simulink library "lib_all"  (Simulink 7.6)
settings: Solver=ode45  SolverMode=SingleTasking  StartTime=0.0  StopTime=10.0  RelTol=1e-3
values from neighbouring files (symbols this model uses):
  map_lib_03 = [6.8679 7.0159 7.4571 8.1849 9.1892 10.456 11.97 13.71 15.655 17.781 20.063 22.474 ... (803 elements, 73x11)]    [scr_simdata.mat]    (also in scr_simdata_all.mat)
  map_lib_06 = [2.5784 2.6727 2.9588 3.4453 4.1444 5.068 6.226 7.6242 9.2634 11.139 13.24 15.552 ... (803 elements, 73x11)]    [scr_simdata.mat]    (also in scr_simdata_all.mat)
  map_lib_09 = [0.0025784 0.0026727 0.0029588 0.0034453 0.0041444 0.005068 0.006226 0.0076242 0.0092634 0.011139 0.01324 0.015552 ... (803 elements, 73x11)]    [scr_simdata.mat]    (also in scr_simdata_all.mat)
  map_lib_10 = [2.74 2.75 2.76 2.77 2.78 2.79 2.8 2.81 2.82 2.83 2.84 2.85 ... (81 elements, 1x81)]    [scr_simdata.mat]    (also in scr_simdata_all.mat)
  map_lib_11 = [1.1983 1.2027 1.207 1.2113 1.2156 1.2198 1.2241 1.2283 1.2326 1.2368 1.241 1.2451 ... (81 elements, 1x81)]    [scr_simdata.mat]    (also in scr_simdata_all.mat)
  map_lib_12 = [2.74 2.75 2.76 2.77 2.78 2.79 2.8 2.81 2.82 2.83 2.84 2.85 ... (81 elements, 1x81)]    [scr_simdata.mat]    (also in scr_simdata_all.mat)
  map_lib_13 = [1.74 1.75 1.76 1.77 1.78 1.79 1.8 1.81 1.82 1.83 1.84 1.85 ... (81 elements, 1x81)]    [scr_simdata.mat]    (also in scr_simdata_all.mat)
  map_lib_16 = [0.00048828125 1.6159 1.8962 2.0821 2.2251 2.3426 2.4433 2.5318 2.611 2.683 2.749 2.8101 ... (1419 elements, 129x11)]    [scr_simdata.mat]    (also in scr_simdata_all.mat)
  map_mr2_01 = [0 800 1600 2400 3200 4000]    [scr_simdata.mat]    (also in scr_simdata_all.mat)
  map_mr2_02 = [1.32 1.32 1.33 1.34 1.36 1.38]    [scr_simdata.mat]    (also in scr_simdata_all.mat)
  map_mr2_03 = [0 800 1600 2400 3200 4000]    [scr_simdata.mat]    (also in scr_simdata_all.mat)
  map_mr2_04 = [1.32 1.32 1.33 1.34 1.36 1.38]    [scr_simdata.mat]    (also in scr_simdata_all.mat)
  map_mr2_05 = [0 800 1600 2400 3200 4000]    [scr_simdata.mat]    (also in scr_simdata_all.mat)
  map_mr2_06 = [1.32 1.32 1.33 1.34 1.36 1.38]    [scr_simdata.mat]    (also in scr_simdata_all.mat)
  Parameter1 = 6    [scr_simdata.mat]    (also in scr_simdata_all.mat)
  Parameter101 = 30    [scr_simdata.mat]    (also in scr_simdata_all.mat)
  Parameter102 = 12    [scr_simdata.mat]    (also in scr_simdata_all.mat)
  Parameter103 = -240    [scr_simdata.mat]    (also in scr_simdata_all.mat)
  Parameter108 = 48    [scr_simdata.mat]    (also in scr_simdata_all.mat)
  Parameter120 = 16    [scr_simdata.mat]    (also in scr_simdata_all.mat)
  Parameter121 = 87    [scr_simdata.mat]    (also in scr_simdata_all.mat)
  Parameter123 = 78    [scr_simdata.mat]    (also in scr_simdata_all.mat)
  Parameter124 = 60    [scr_simdata.mat]    (also in scr_simdata_all.mat)
  Parameter125 = -480    [scr_simdata.mat]    (also in scr_simdata_all.mat)
  Parameter130 = 96    [scr_simdata.mat]    (also in scr_simdata_all.mat)
  Parameter131 = 3    [scr_simdata.mat]    (also in scr_simdata_all.mat)
  Parameter144 = 3    [scr_simdata.mat]    (also in scr_simdata_all.mat)
  Parameter18 = 128    [scr_simdata.mat]    (also in scr_simdata_all.mat)
  Parameter19 = 16    [scr_simdata.mat]    (also in scr_simdata_all.mat)
  Parameter2 = 12    [scr_simdata.mat]    (also in scr_simdata_all.mat)
  Parameter20 = 7    [scr_simdata.mat]    (also in scr_simdata_all.mat)
  Parameter21 = 4    [scr_simdata.mat]    (also in scr_simdata_all.mat)
  Parameter3 = 18    [scr_simdata.mat]    (also in scr_simdata_all.mat)
  Parameter32 = 1    [scr_simdata.mat]    (also in scr_simdata_all.mat)
  Parameter34 = 24    [scr_simdata.mat]    (also in scr_simdata_all.mat)
  Parameter4 = 138    [scr_simdata.mat]    (also in scr_simdata_all.mat)
  Parameter42 = 48    [scr_simdata.mat]    (also in scr_simdata_all.mat)
  Parameter5 = 135    [scr_simdata.mat]    (also in scr_simdata_all.mat)
  Parameter55 = 0    [scr_simdata.mat]    (also in scr_simdata_all.mat)
  Parameter56 = 0    [scr_simdata.mat]    (also in scr_simdata_all.mat)
  Parameter57 = 1    [scr_simdata.mat]    (also in scr_simdata_all.mat)
  Parameter58 = 1    [scr_simdata.mat]    (also in scr_simdata_all.mat)
  Parameter59 = 2    [scr_simdata.mat]    (also in scr_simdata_all.mat)
  Parameter6 = 132    [scr_simdata.mat]    (also in scr_simdata_all.mat)
  Parameter60 = 2    [scr_simdata.mat]    (also in scr_simdata_all.mat)
  Parameter63 = 144    [scr_simdata.mat]    (also in scr_simdata_all.mat)
  Parameter7 = 126    [scr_simdata.mat]    (also in scr_simdata_all.mat)
  Parameter76 = 64    [scr_simdata.mat]    (also in scr_simdata_all.mat)
  Parameter77 = 135    [scr_simdata.mat]    (also in scr_simdata_all.mat)
  Parameter79 = 126    [scr_simdata.mat]    (also in scr_simdata_all.mat)
  Parameter80 = 108    [scr_simdata.mat]    (also in scr_simdata_all.mat)
  Parameter81 = 0    [scr_simdata.mat]    (also in scr_simdata_all.mat)
  Parameter86 = 0    [scr_simdata.mat]    (also in scr_simdata_all.mat)
  Parameter98 = 112    [scr_simdata.mat]    (also in scr_simdata_all.mat)
  Parameter99 = 39    [scr_simdata.mat]    (also in scr_simdata_all.mat)
undefined symbols (not defined in the file or in its folder): cylvolkp_hih_map2h, cylvolkp_map2h, pk1k_map2h
libraries: simulink x2
(part 13 of 36)

== system lib_all/SubSystem176/SubSystem178/SubSystem182/SubSystem236 ==
  note: Text41
  Block535 : Inport
  Block536 : Inport  Port=2
  Block537 : Inport  Port=3
  Block538 : Inport  Port=4
  Block539 : Constant  Value=0  OutDataTypeStr=double  SampleTime=-1
  Block540 : Constant  OutDataTypeStr=double  SampleTime=-1
  Block541 : Constant  Value=0  OutDataTypeStr=double  SampleTime=-1
  Block542 : Gain  Gain=1/5  SaturateOnIntegerOverflow=on  OutDataTypeStr=Inherit: Same as input  ParamDataTypeStr=Inherit: Same as input
      settings: AttributesFormatString=Attribute119
  Block543 : Product  OutDataTypeStr=double  SaturateOnIntegerOverflow=on  RndMeth=Zero  InputSameDT=on
  Block544 : Sum  Inputs=-+  OutDataTypeStr=double  RndMeth=Zero  SaturateOnIntegerOverflow=on  InputSameDT=on
  Block545 : Sum  OutDataTypeStr=double  RndMeth=Zero  SaturateOnIntegerOverflow=on  InputSameDT=on
  Block546 : Sum  OutDataTypeStr=double  RndMeth=Zero  SaturateOnIntegerOverflow=on  InputSameDT=on
  Block547 : Switch  Threshold=0.5  RndMeth=Zero  SaturateOnIntegerOverflow=on  InputSameDT=on
  SubSystem238 : Subsystem
  SubSystem239 : Subsystem
  SubSystem245 : Subsystem
  Block656 : Outport
  wires:
  Block546/1 -> SubSystem245/3(Block577), SubSystem239/2(Block549)
  Block535/1 -> Block546/1
  Block542/1 -> Block545/2
  Block543/1 -> Block542/1
  Block540/1 -> Block546/2
  Block539/1 -> Block547/1
  Block547/1 -> Block656/1
  SubSystem238/1(Out1) -> Block547/2
  Block541/1 -> SubSystem238/2(In2)
  Block537/1 -> SubSystem239/3(Block550)
  Block545/1 -> SubSystem238/1(In1), Block547/3
  SubSystem239/2(Block574) -> Block543/2
  Block544/1 -> Block543/1  "SigLabel14"
  SubSystem245/2(Block655) -> Block544/2
  SubSystem245/1(Block654) -> Block544/1, Block545/1
  Block538/1 -> SubSystem245/2(Block576)
  SubSystem239/1(Block573) -> SubSystem245/1(Block575)
  Block536/1 -> SubSystem239/1(Block548)

== system lib_all/SubSystem176/SubSystem178/SubSystem182/SubSystem289 ==
  note: Text42
  Block657 : Inport
  Block658 : Inport  Port=2
  Block659 : Inport  Port=3
  Block660 : SwitchCase  CaseConditions={1 2 3}
  Block661 : Terminator
  SubSystem290 : Subsystem
      settings: RTWSystemCode=Inline
  SubSystem292 : Subsystem
      settings: RTWSystemCode=Inline
  SubSystem294 : Subsystem
      settings: RTWSystemCode=Inline
  wires:
  Block660/2 -> SubSystem292/Action
  Block660/1 -> SubSystem290/Action
  Block659/1 -> SubSystem292/2(Block666), SubSystem294/2(Block669), SubSystem290/2(Block663)
  Block657/1 -> SubSystem292/1(Block665), SubSystem294/1(Block668), SubSystem290/1(Block662)
  Block658/1 -> Block660/1
  Block660/4 -> Block661/1
  Block660/3 -> SubSystem294/Action

== system lib_all/SubSystem176/SubSystem178/SubSystem182/SubSystem296 ==
  note: Text43
  Block671 : Inport
  Block672 : Inport  Port=2
  Block673 : Inport  Port=3
  Block674 : SwitchCase  CaseConditions={1 2 3}
  Block675 : Terminator
  SubSystem297 : Subsystem
      settings: RTWSystemCode=Inline
  SubSystem299 : Subsystem
      settings: RTWSystemCode=Inline
  SubSystem301 : Subsystem
      settings: RTWSystemCode=Inline
  wires:
  Block672/1 -> Block674/1
  Block671/1 -> SubSystem297/1(Block676), SubSystem299/1(Block679), SubSystem301/1(Block682)
  Block673/1 -> SubSystem297/2(Block677), SubSystem299/2(Block680), SubSystem301/2(Block683)
  Block674/1 -> SubSystem297/Action
  Block674/2 -> SubSystem299/Action
  Block674/4 -> Block675/1
  Block674/3 -> SubSystem301/Action

== system lib_all/SubSystem176/SubSystem303/SubSystem305/SubSystem306 ==
  note: ***編集禁止***
  N : Inport
  For_loop : Stateflow chart  TreatAsAtomicUnit=on
  action : Outport
  i : Outport  Port=2
  wires:
  For_loop/2(function_call) -> action/1
  N/1 -> For_loop/1(N_Loop)
  For_loop/1(idx) -> i/1

== system lib_all/SubSystem176/SubSystem303/SubSystem305/SubSystem308 ==
  Block695 : Inport
  Block696 : Inport  Port=2
  Block697 : Inport  Port=3
  Block698 : Constant  Value=Parameter21  OutDataTypeStr=double  SampleTime=-1
  Block699 : Constant  Value=2  OutDataTypeStr=double  SampleTime=-1
  Block700 : Constant  Value=0  OutDataTypeStr=double  SampleTime=-1
  Block701 : Math  Operator=pow  SaturateOnIntegerOverflow=on
  Block702 : Product  Inputs=*/  OutDataTypeStr=double  SaturateOnIntegerOverflow=on  RndMeth=Zero  InputSameDT=on
  Block703 : Product  Inputs=*/  OutDataTypeStr=double  SaturateOnIntegerOverflow=on  RndMeth=Zero  InputSameDT=on
  Block704 : Sum  Inputs=-+  OutDataTypeStr=double  RndMeth=Zero  SaturateOnIntegerOverflow=on  InputSameDT=on
  SubSystem309 : Subsystem
  SubSystem310 : Subsystem  Priority=2  TreatAsAtomicUnit=on  mask(***ATC.DSWVEC***){bd_version=1.01.A, r0=DSM377, r1=-1, r2=, r3=, r4=, r5=, r6=, r7=, r8=, r9=, c0=, c1=, c2=, c3=, c4=, c5=, c6=, c7=, c8=, c9=}
      mask prompts: bd_version "Version"
      mask help: これはAutoCからのブロックです．編集禁止．
      settings: AttributesFormatString=Attribute141  OpenFcn=warndlg('変更禁止!');
      mask Help: web(ui_blockhelpmng(gcb));
      mask Display: disp('ATC.DSRVEC')
  SubSystem311 : Subsystem  Priority=3  TreatAsAtomicUnit=on  mask(***ATC.DSWVEC***){bd_version=1.01.A, r0=DSM379, r1=-1, r2=, r3=, r4=, r5=, r6=, r7=, r8=, r9=, c0=, c1=, c2=, c3=, c4=, c5=, c6=, c7=, c8=, c9=}
      mask prompts: bd_version "Version"
      mask help: これはAutoCからのブロックです．編集禁止．
      settings: AttributesFormatString=Attribute142  OpenFcn=warndlg('変更禁止!');
      mask Help: web(ui_blockhelpmng(gcb));
      mask Display: disp('ATC.DSRVEC')
  SubSystem312 : Subsystem  Priority=1  TreatAsAtomicUnit=on  mask(***ATC.DSWVEC***){bd_version=1.01.A, r0=DSM381, r1=-1, r2=, r3=, r4=, r5=, r6=, r7=, r8=, r9=, c0=, c1=, c2=, c3=, c4=, c5=, c6=, c7=, c8=, c9=}
      mask prompts: bd_version "Version"
      mask help: これはAutoCからのブロックです．編集禁止．
      settings: AttributesFormatString=Attribute143  OpenFcn=warndlg('変更禁止!');
      mask Help: web(ui_blockhelpmng(gcb));
      mask Display: disp('ATC.DSRVEC')
  SubSystem313 : Subsystem  Priority=4
      settings: AttributesFormatString=Attribute144
  wires:
  Block699/1 -> Block701/1
  Block698/1 -> Block701/2
  Block702/1 -> SubSystem312/1(input), Block704/2
  Block703/1 -> SubSystem310/1(input), Block704/1
  Block695/1 -> Block702/1
  Block697/1 -> SubSystem312/2(element), SubSystem311/2(element), SubSystem310/2(element)
  Block696/1 -> Block703/1
  Block704/1 -> SubSystem311/1(input), SubSystem309/1(In1)
  Block700/1 -> SubSystem309/2(In2)
  SubSystem309/1(Out1) -> SubSystem313/Enable
  Block701/1 -> Block702/2, Block703/2

== system lib_all/SubSystem176/SubSystem303/SubSystem305/SubSystem318 ==
  Block715 : Inport
  Block716 : Inport  Port=2
  Block717 : TriggerPort  TriggerType=function-call
  Block718 : Constant  OutDataTypeStr=double  SampleTime=-1
  Block719 : Sum  OutDataTypeStr=double  RndMeth=Zero  SaturateOnIntegerOverflow=on  InputSameDT=on
  Block720 : Sum  OutDataTypeStr=double  RndMeth=Zero  SaturateOnIntegerOverflow=on  InputSameDT=on
  Block721 : Switch  Threshold=0.5  RndMeth=Zero  SaturateOnIntegerOverflow=on  InputSameDT=on
  Block722 : Switch  Threshold=0.5  RndMeth=Zero  SaturateOnIntegerOverflow=on  InputSameDT=on
  Block723 : UnitDelay  SampleTime=-1
  Block724 : UnitDelay  SampleTime=-1
  SubSystem319 : Subsystem
  SubSystem320 : Subsystem
  SubSystem324 : Subsystem
  Block733 : Outport
  Block734 : Outport  Port=2
  wires:
  Block715/1 -> SubSystem324/2(Block730), SubSystem320/2(Block726), SubSystem319/1(In1)
  Block718/1 -> SubSystem319/2(In2)
  SubSystem319/1(Out1) -> Block721/2, Block722/2
  Block721/1 -> Block733/1, Block723/1
  Block716/1 -> SubSystem324/1(Block729), SubSystem320/1(Block725)
  Block719/1 -> Block721/3
  SubSystem324/1(Block732) -> Block719/1, Block721/1
  Block723/1 -> Block719/2
  Block722/1 -> Block734/1, Block724/1
  Block720/1 -> Block722/3
  SubSystem320/1(Block728) -> Block720/1, Block722/1
  Block724/1 -> Block720/2

== system lib_all/SubSystem176/SubSystem329/SubSystem332/SubSystem333 ==
  note: ***編集禁止***
  N : Inport
  For_loop : Stateflow chart  TreatAsAtomicUnit=on
  action : Outport
  i : Outport  Port=2
  wires:
  For_loop/2(function_call) -> action/1
  N/1 -> For_loop/1(N_Loop)
  For_loop/1(idx) -> i/1

== system lib_all/SubSystem176/SubSystem329/SubSystem332/SubSystem335 ==
  note: ***編集禁止***
  N : Inport
  For_loop : Stateflow chart  TreatAsAtomicUnit=on
  action : Outport
  i : Outport  Port=2
  wires:
  For_loop/2(function_call) -> action/1
  N/1 -> For_loop/1(N_Loop)
  For_loop/1(idx) -> i/1

== system lib_all/SubSystem176/SubSystem329/SubSystem332/SubSystem337 ==
  Block747 : Inport
  Block748 : TriggerPort  TriggerType=function-call
  Block749 : Constant  OutDataTypeStr=double  SampleTime=-1
  Block750 : Product  Inputs=*/  OutDataTypeStr=double  SaturateOnIntegerOverflow=on  RndMeth=Zero  InputSameDT=on
  SubSystem338 : Subsystem  TreatAsAtomicUnit=on  mask(***ATC.DSWVEC***){bd_version=1.01.A, r0=DSM87, r1=-1, r2=, r3=, r4=, r5=, r6=, r7=, r8=, r9=, c0=, c1=, c2=, c3=, c4=, c5=, c6=, c7=, c8=, c9=}
      mask prompts: bd_version "Version"
      mask help: これはAutoCからのブロックです．編集禁止．
      settings: OpenFcn=warndlg('変更禁止!');
      mask Help: web(ui_blockhelpmng(gcb));
      mask Display: disp('ATC.DSRVEC')
  SubSystem339 : Subsystem  TreatAsAtomicUnit=on  mask(***ATC.DSRVEC***){bd_version=1.01.A, r0=DSM379, r1=-1, r2=, r3=, r4=, r5=, r6=, r7=, r8=, r9=, c0=, c1=, c2=, c3=, c4=, c5=, c6=, c7=, c8=, c9=}
      mask prompts: bd_version "Version"
      mask help: これはAutoCからのブロックです．編集禁止．
      settings: OpenFcn=warndlg('変更禁止!');
      mask Help: web(ui_blockhelpmng(gcb));
      mask Display: disp('ATC.DSRVEC')
  SubSystem340 : Subsystem  TreatAsAtomicUnit=on  mask(***ATC.DSRVEC***){bd_version=1.01.A, r0=DSM379, r1=-1, r2=, r3=, r4=, r5=, r6=, r7=, r8=, r9=, c0=, c1=, c2=, c3=, c4=, c5=, c6=, c7=, c8=, c9=}
      mask prompts: bd_version "Version"
      mask help: これはAutoCからのブロックです．編集禁止．
      settings: OpenFcn=warndlg('変更禁止!');
      mask Help: web(ui_blockhelpmng(gcb));
      mask Display: disp('ATC.DSRVEC')
  wires:
  Block749/1 -> SubSystem340/1(element)
  Block747/1 -> SubSystem338/2(element), SubSystem339/1(element)
  SubSystem340/1(Output) -> Block750/1
  SubSystem339/1(Output) -> Block750/2
  Block750/1 -> SubSystem338/1(input)

== system lib_all/SubSystem176/SubSystem329/SubSystem332/SubSystem341 ==
  Block751 : Inport
  Block752 : TriggerPort  TriggerType=function-call
  Block753 : Constant  Value=0  OutDataTypeStr=double  SampleTime=-1
  SubSystem342 : Subsystem  TreatAsAtomicUnit=on  mask(***ATC.DSWVEC***){bd_version=1.01.A, r0=DSM378, r1=-1, r2=, r3=, r4=, r5=, r6=, r7=, r8=, r9=, c0=, c1=, c2=, c3=, c4=, c5=, c6=, c7=, c8=, c9=}
      mask prompts: bd_version "Version"
      mask help: これはAutoCからのブロックです．編集禁止．
      settings: OpenFcn=warndlg('変更禁止!');
      mask Help: web(ui_blockhelpmng(gcb));
      mask Display: disp('ATC.DSRVEC')
  wires:
  Block751/1 -> SubSystem342/2(element)
  Block753/1 -> SubSystem342/1(input)

== system lib_all/SubSystem176/SubSystem329/SubSystem332/SubSystem343 ==
  Block754 : Constant  Value=0  OutDataTypeStr=double  SampleTime=-1
  Block755 : DataStoreWrite  DataStoreName=DSM365
  wires:
  Block754/1 -> Block755/1

== system lib_all/SubSystem1770/SubSystem1774/SubSystem1778/SubSystem1779 ==
  note: Text349
  Block4857 : Inport
  Block4858 : Inport  Port=2
  Block4859 : Constant  Value=5  OutDataTypeStr=double  SampleTime=-1
  Block4860 : DataStoreRead  DataStoreName=Pair
  Block4861 : Gain  Gain=1/5  SaturateOnIntegerOverflow=on  OutDataTypeStr=Inherit: Same as input  ParamDataTypeStr=Inherit: Same as input
      settings: AttributesFormatString=Attribute955
  Block4865 : Sum  Inputs=+-  OutDataTypeStr=double  RndMeth=Zero  SaturateOnIntegerOverflow=on  InputSameDT=on
  SingleForIterator : Subsystem  TreatAsAtomicUnit=on
      settings: RTWSystemCode=Inline
  SubSystem1783 : Subsystem
  Block4890 : Outport
  wires:
  SingleForIterator/2(i) -> SubSystem1783/1(Block4866)
  SingleForIterator/1(action) -> SubSystem1783/Trigger
  Block4859/1 -> SingleForIterator/1(N)
  Block4857/1 -> SubSystem1783/2(Block4867)
  Block4858/1 -> SubSystem1783/3(Block4868)
  SubSystem1783/1(Block4889) -> Block4861/1
  Block4860/1 -> Block4865/1
  Block4865/1 -> Block4890/1
  Block4861/1 -> Block4865/2

== system lib_all/SubSystem1770/SubSystem1774/SubSystem1778/SubSystem1790 ==
  note: ***編集禁止***
  input : Inport
  element : Inport  Port=2
  Assignment : Assignment  IndexOptions=Index vector (port)  Indices=1  OutputSizes=[1 1]
  Inside_DSR : DataStoreRead  DataStoreName=DSM1
  Inside_DSW : DataStoreWrite  DataStoreName=DSM1
  wires:
  element/1 -> Assignment/3
  input/1 -> Assignment/2
  Inside_DSR/1 -> Assignment/1
  Assignment/1 -> Inside_DSW/1

== system lib_all/SubSystem1770/SubSystem1774/SubSystem1791/SubSystem1792 ==
  note: Text352
  Block4896 : Inport
  Block4897 : Inport  Port=2
  Block4898 : Constant  Value=5  OutDataTypeStr=double  SampleTime=-1
  Block4899 : DataStoreRead  DataStoreName=Pair
  Block4900 : Gain  Gain=1/5  SaturateOnIntegerOverflow=on  OutDataTypeStr=Inherit: Same as input  ParamDataTypeStr=Inherit: Same as input
      settings: AttributesFormatString=Attribute967
  Block4904 : Sum  Inputs=+-  OutDataTypeStr=double  RndMeth=Zero  SaturateOnIntegerOverflow=on  InputSameDT=on
  SubSystem1794 : Subsystem  TreatAsAtomicUnit=on
      settings: RTWSystemCode=Inline
  SubSystem1796 : Subsystem
  Block4929 : Outport
  wires:
  SubSystem1794/2(i) -> SubSystem1796/1(Block4905)
  SubSystem1794/1(action) -> SubSystem1796/Trigger
  Block4898/1 -> SubSystem1794/1(N)
  Block4896/1 -> SubSystem1796/2(Block4906)
  Block4897/1 -> SubSystem1796/3(Block4907)
  SubSystem1796/1(Block4928) -> Block4900/1
  Block4899/1 -> Block4904/1
  Block4904/1 -> Block4929/1
  Block4900/1 -> Block4904/2

== system lib_all/SubSystem1770/SubSystem1774/SubSystem1791/SubSystem1803 ==
  note: ***編集禁止***
  input : Inport
  element : Inport  Port=2
  Assignment : Assignment  IndexOptions=Index vector (port)  Indices=1  OutputSizes=[1 1]
  Inside_DSR : DataStoreRead  DataStoreName=DSM1
  Inside_DSW : DataStoreWrite  DataStoreName=DSM1
  wires:
  element/1 -> Assignment/3
  input/1 -> Assignment/2
  Inside_DSR/1 -> Assignment/1
  Assignment/1 -> Inside_DSW/1

== system lib_all/SubSystem1770/SubSystem1774/SubSystem1804/SubSystem1805 ==
  note: Text355
  Block4935 : Inport
  Block4936 : Inport  Port=2
  Block4937 : Constant  Value=5  OutDataTypeStr=double  SampleTime=-1
  Block4938 : DataStoreRead  DataStoreName=Pair
  Block4939 : Gain  Gain=1/5  SaturateOnIntegerOverflow=on  OutDataTypeStr=Inherit: Same as input  ParamDataTypeStr=Inherit: Same as input
      settings: AttributesFormatString=Attribute979
  Block4943 : Sum  Inputs=+-  OutDataTypeStr=double  RndMeth=Zero  SaturateOnIntegerOverflow=on  InputSameDT=on
  SubSystem1807 : Subsystem  TreatAsAtomicUnit=on
      settings: RTWSystemCode=Inline
  SubSystem1809 : Subsystem
  Block4968 : Outport
  wires:
  SubSystem1807/2(i) -> SubSystem1809/1(Block4944)
  SubSystem1807/1(action) -> SubSystem1809/Trigger
  Block4937/1 -> SubSystem1807/1(N)
  Block4935/1 -> SubSystem1809/2(Block4945)
  Block4936/1 -> SubSystem1809/3(Block4946)
  SubSystem1809/1(Block4967) -> Block4939/1
  Block4938/1 -> Block4943/1
  Block4943/1 -> Block4968/1
  Block4939/1 -> Block4943/2

== system lib_all/SubSystem1770/SubSystem1774/SubSystem1804/SubSystem1816 ==
  note: ***編集禁止***
  input : Inport
  element : Inport  Port=2
  Assignment : Assignment  IndexOptions=Index vector (port)  Indices=1  OutputSizes=[1 1]
  Inside_DSR : DataStoreRead  DataStoreName=DSM1
  Inside_DSW : DataStoreWrite  DataStoreName=DSM1
  wires:
  element/1 -> Assignment/3
  input/1 -> Assignment/2
  Inside_DSR/1 -> Assignment/1
  Assignment/1 -> Inside_DSW/1

== system lib_all/SubSystem1770/SubSystem1817/SubSystem1821/SubSystem1822 ==
  note: Text358
  Block4983 : Inport
  Block4984 : Inport  Port=2
  Block4985 : Constant  Value=5  OutDataTypeStr=double  SampleTime=-1
  Block4986 : DataStoreRead  DataStoreName=Pair
  Block4987 : Gain  Gain=1/5  SaturateOnIntegerOverflow=on  OutDataTypeStr=Inherit: Same as input  ParamDataTypeStr=Inherit: Same as input
      settings: AttributesFormatString=Attribute996
  Block4991 : Sum  Inputs=+-  OutDataTypeStr=double  RndMeth=Zero  SaturateOnIntegerOverflow=on  InputSameDT=on
  SubSystem1824 : Subsystem  TreatAsAtomicUnit=on
      settings: RTWSystemCode=Inline
  SubSystem1826 : Subsystem
  Block5016 : Outport
  wires:
  SubSystem1824/2(i) -> SubSystem1826/1(Block4992)
  SubSystem1824/1(action) -> SubSystem1826/Trigger
  Block4985/1 -> SubSystem1824/1(N)
  Block4983/1 -> SubSystem1826/2(Block4993)
  Block4984/1 -> SubSystem1826/3(Block4994)
  SubSystem1826/1(Block5015) -> Block4987/1
  Block4986/1 -> Block4991/1
  Block4991/1 -> Block5016/1
  Block4987/1 -> Block4991/2

== system lib_all/SubSystem1770/SubSystem1817/SubSystem1821/SubSystem1833 ==
  note: ***編集禁止***
  input : Inport
  element : Inport  Port=2
  Assignment : Assignment  IndexOptions=Index vector (port)  Indices=1  OutputSizes=[1 1]
  Inside_DSR : DataStoreRead  DataStoreName=DSM1
  Inside_DSW : DataStoreWrite  DataStoreName=DSM1
  wires:
  element/1 -> Assignment/3
  input/1 -> Assignment/2
  Inside_DSR/1 -> Assignment/1
  Assignment/1 -> Inside_DSW/1

== system lib_all/SubSystem1770/SubSystem1817/SubSystem1834/SubSystem1835 ==
  note: Text361
  Block5022 : Inport
  Block5023 : Inport  Port=2
  Block5024 : Constant  Value=5  OutDataTypeStr=double  SampleTime=-1
  Block5025 : DataStoreRead  DataStoreName=Pair
  Block5026 : Gain  Gain=1/5  SaturateOnIntegerOverflow=on  OutDataTypeStr=Inherit: Same as input  ParamDataTypeStr=Inherit: Same as input
      settings: AttributesFormatString=Attribute1008
  Block5030 : Sum  Inputs=+-  OutDataTypeStr=double  RndMeth=Zero  SaturateOnIntegerOverflow=on  InputSameDT=on
  SubSystem1837 : Subsystem  TreatAsAtomicUnit=on
      settings: RTWSystemCode=Inline
  SubSystem1839 : Subsystem
  Block5055 : Outport
  wires:
  SubSystem1837/2(i) -> SubSystem1839/1(Block5031)
  SubSystem1837/1(action) -> SubSystem1839/Trigger
  Block5024/1 -> SubSystem1837/1(N)
  Block5022/1 -> SubSystem1839/2(Block5032)
  Block5023/1 -> SubSystem1839/3(Block5033)
  SubSystem1839/1(Block5054) -> Block5026/1
  Block5025/1 -> Block5030/1
  Block5030/1 -> Block5055/1
  Block5026/1 -> Block5030/2

== system lib_all/SubSystem1770/SubSystem1817/SubSystem1834/SubSystem1846 ==
  note: ***編集禁止***
  input : Inport
  element : Inport  Port=2
  Assignment : Assignment  IndexOptions=Index vector (port)  Indices=1  OutputSizes=[1 1]
  Inside_DSR : DataStoreRead  DataStoreName=DSM1
  Inside_DSW : DataStoreWrite  DataStoreName=DSM1
  wires:
  element/1 -> Assignment/3
  input/1 -> Assignment/2
  Inside_DSR/1 -> Assignment/1
  Assignment/1 -> Inside_DSW/1

== system lib_all/SubSystem1770/SubSystem1817/SubSystem1847/SubSystem1848 ==
  note: Text364
  Block5061 : Inport
  Block5062 : Inport  Port=2
  Block5063 : Constant  Value=5  OutDataTypeStr=double  SampleTime=-1
  Block5064 : DataStoreRead  DataStoreName=Pair
  Block5065 : Gain  Gain=1/5  SaturateOnIntegerOverflow=on  OutDataTypeStr=Inherit: Same as input  ParamDataTypeStr=Inherit: Same as input
      settings: AttributesFormatString=Attribute1020
  Block5069 : Sum  Inputs=+-  OutDataTypeStr=double  RndMeth=Zero  SaturateOnIntegerOverflow=on  InputSameDT=on
  SubSystem1850 : Subsystem  TreatAsAtomicUnit=on
      settings: RTWSystemCode=Inline
  SubSystem1852 : Subsystem
  Block5094 : Outport
  wires:
  SubSystem1850/2(i) -> SubSystem1852/1(Block5070)
  SubSystem1850/1(action) -> SubSystem1852/Trigger
  Block5063/1 -> SubSystem1850/1(N)
  Block5061/1 -> SubSystem1852/2(Block5071)
  Block5062/1 -> SubSystem1852/3(Block5072)
  SubSystem1852/1(Block5093) -> Block5065/1
  Block5064/1 -> Block5069/1
  Block5069/1 -> Block5094/1
  Block5065/1 -> Block5069/2

== system lib_all/SubSystem1770/SubSystem1817/SubSystem1847/SubSystem1859 ==
  note: ***編集禁止***
  input : Inport
  element : Inport  Port=2
  Assignment : Assignment  IndexOptions=Index vector (port)  Indices=1  OutputSizes=[1 1]
  Inside_DSR : DataStoreRead  DataStoreName=DSM1
  Inside_DSW : DataStoreWrite  DataStoreName=DSM1
  wires:
  element/1 -> Assignment/3
  input/1 -> Assignment/2
  Inside_DSR/1 -> Assignment/1
  Assignment/1 -> Inside_DSW/1

== system lib_all/SubSystem1860/SubSystem1864/SubSystem1867/SubSystem1868 ==
  note: ***編集禁止***
  element : Inport
  Inside_DSR : DataStoreRead  DataStoreName=DSM7
  Selector : Selector  IndexOptions=Index vector (port)  Indices=[1 3]  OutputSizes=1
  Output : Outport
  wires:
  Selector/1 -> Output/1
  element/1 -> Selector/2
  Inside_DSR/1 -> Selector/1

== system lib_all/SubSystem1860/SubSystem1864/SubSystem1867/SubSystem1869 ==
  note: ***編集禁止***
  element : Inport
  Inside_DSR : DataStoreRead  DataStoreName=DSM6
  Selector : Selector  IndexOptions=Index vector (port)  Indices=[1 3]  OutputSizes=1
  Output : Outport
  wires:
  Inside_DSR/1 -> Selector/1
  element/1 -> Selector/2
  Selector/1 -> Output/1

== system lib_all/SubSystem1860/SubSystem1864/SubSystem1867/SubSystem1870 ==
  note: ***編集禁止***
  element : Inport
  Inside_DSR : DataStoreRead  DataStoreName=DSM19
  Selector : Selector  IndexOptions=Index vector (port)  Indices=[1 3]  OutputSizes=1
  Output : Outport
  wires:
  Selector/1 -> Output/1
  element/1 -> Selector/2
  Inside_DSR/1 -> Selector/1

== system lib_all/SubSystem1879/SubSystem1881/SubSystem1882/Product ==
  note: ***編集禁止***
  mult : Inport
  div : Inport  Port=2
  init : Inport  Port=3
  Constant1 : Constant  OutDataTypeStr=Inherit: Inherit via back propagation
  Constant2 : Constant  Value=0  OutDataTypeStr=Inherit: Inherit via back propagation
  Product : Product  Inputs=*/  SaturateOnIntegerOverflow=on  RndMeth=Zero  InputSameDT=on  OutDataTypeStr=Inherit: Same as first input
  Relational Operator : RelationalOperator  Operator=~=  InputSameDT=on
  Switch : Switch  Threshold=0.5  SaturateOnIntegerOverflow=on  InputSameDT=on
  Switch1 : Switch  Threshold=0.5  SaturateOnIntegerOverflow=on  InputSameDT=on
  Out : Outport
  wires:
  mult/1 -> Product/1
  Product/1 -> Switch/1
  Relational Operator/1 -> Switch/2, Switch1/2
  init/1 -> Switch/3
  Switch/1 -> Out/1
  div/1 -> Switch1/1, Relational Operator/1
  Switch1/1 -> Product/2
  Constant2/1 -> Relational Operator/2
  Constant1/1 -> Switch1/3

== system lib_all/SubSystem1879/SubSystem1881/SubSystem1884/SubSystem1885 ==
  note: ***編集禁止***
  In1 : Inport
  In2 : Inport  Port=2
  RO1 : RelationalOperator  Operator=>  InputSameDT=on
  single1 : DataTypeConversion  OutDataTypeStr=single  SaturateOnIntegerOverflow=on  RndMeth=Zero
  single2 : DataTypeConversion  OutDataTypeStr=single  SaturateOnIntegerOverflow=on  RndMeth=Zero
  Out1 : Outport
  wires:
  RO1/1 -> Out1/1
  In2/1 -> single2/1
  In1/1 -> single1/1
  single1/1 -> RO1/1
  single2/1 -> RO1/2

== system lib_all/SubSystem1879/SubSystem1881/SubSystem1884/SubSystem1886 ==
  note: ***編集禁止***
  In1 : Inport
  In2 : Inport  Port=2
  LO1 : Logic
  RO1 : RelationalOperator  Operator=<  InputSameDT=on
  RO2 : RelationalOperator  Operator=~=  InputSameDT=on
  single1 : DataTypeConversion  OutDataTypeStr=single  SaturateOnIntegerOverflow=on  RndMeth=Zero
  single2 : DataTypeConversion  OutDataTypeStr=single  SaturateOnIntegerOverflow=on  RndMeth=Zero
  Out1 : Outport
  wires:
  In2/1 -> single2/1
  In1/1 -> single1/1
  single1/1 -> RO1/1, RO2/1
  single2/1 -> RO1/2, RO2/2
  RO1/1 -> LO1/1
  RO2/1 -> LO1/2
  LO1/1 -> Out1/1

== system lib_all/SubSystem1879/SubSystem1887/SubSystem1891/SubSystem1892 ==
  Block5195 : Inport
  Block5196 : Inport  Port=2
  Block5197 : MultiPortSwitch  zeroidx=off  RndMeth=Zero  SaturateOnIntegerOverflow=on  InputSameDT=on
  SubSystem1893 : Subsystem  TreatAsAtomicUnit=on  mask(***ATC.DSRVEC***){bd_version=1.01.A, r0=DSM18, r1=-1, r2=, r3=, r4=, r5=, r6=, r7=, r8=, r9=, c0=, c1=, c2=, c3=, c4=, c5=, c6=, c7=, c8=, c9=}
      mask prompts: bd_version "Version"
      mask help: これはAutoCからのブロックです．編集禁止．
      settings: OpenFcn=warndlg('変更禁止!');
      mask Help: web(ui_blockhelpmng(gcb));
      mask Display: disp('ATC.DSRVEC')
  SubSystem1894 : Subsystem  TreatAsAtomicUnit=on  mask(***ATC.DSRVEC***){bd_version=1.01.A, r0=DSM8, r1=-1, r2=, r3=, r4=, r5=, r6=, r7=, r8=, r9=, c0=, c1=, c2=, c3=, c4=, c5=, c6=, c7=, c8=, c9=}
      mask prompts: bd_version "Version"
      mask help: これはAutoCからのブロックです．編集禁止．
      settings: OpenFcn=warndlg('変更禁止!');
      mask Help: web(ui_blockhelpmng(gcb));
      mask Display: disp('ATC.DSRVEC')
  SubSystem1895 : Subsystem  TreatAsAtomicUnit=on  mask(***ATC.DSRVEC***){bd_version=1.01.A, r0=DSM11, r1=-1, r2=, r3=, r4=, r5=, r6=, r7=, r8=, r9=, c0=, c1=, c2=, c3=, c4=, c5=, c6=, c7=, c8=, c9=}
      mask prompts: bd_version "Version"
      mask help: これはAutoCからのブロックです．編集禁止．
      settings: OpenFcn=warndlg('変更禁止!');
      mask Help: web(ui_blockhelpmng(gcb));
      mask Display: disp('ATC.DSRVEC')
  Block5198 : Outport
  wires:
  Block5196/1 -> SubSystem1895/1(element), SubSystem1894/1(element), SubSystem1893/1(element)
  SubSystem1895/1(Output) -> Block5197/4
  SubSystem1894/1(Output) -> Block5197/3
  SubSystem1893/1(Output) -> Block5197/2
  Block5197/1 -> Block5198/1
  Block5195/1 -> Block5197/1

== system lib_all/SubSystem1879/SubSystem1887/SubSystem1891/SubSystem1896 ==
  Block5199 : Inport
  Block5200 : Inport  Port=2
  Block5201 : MultiPortSwitch  zeroidx=off  RndMeth=Zero  SaturateOnIntegerOverflow=on  InputSameDT=on
  SubSystem1897 : Subsystem  TreatAsAtomicUnit=on  mask(***ATC.DSRVEC***){bd_version=1.01.A, r0=DSM18, r1=-1, r2=, r3=, r4=, r5=, r6=, r7=, r8=, r9=, c0=, c1=, c2=, c3=, c4=, c5=, c6=, c7=, c8=, c9=}
      mask prompts: bd_version "Version"
      mask help: これはAutoCからのブロックです．編集禁止．
      settings: OpenFcn=warndlg('変更禁止!');
      mask Help: web(ui_blockhelpmng(gcb));
      mask Display: disp('ATC.DSRVEC')
  SubSystem1898 : Subsystem  TreatAsAtomicUnit=on  mask(***ATC.DSRVEC***){bd_version=1.01.A, r0=DSM8, r1=-1, r2=, r3=, r4=, r5=, r6=, r7=, r8=, r9=, c0=, c1=, c2=, c3=, c4=, c5=, c6=, c7=, c8=, c9=}
      mask prompts: bd_version "Version"
      mask help: これはAutoCからのブロックです．編集禁止．
      settings: OpenFcn=warndlg('変更禁止!');
      mask Help: web(ui_blockhelpmng(gcb));
      mask Display: disp('ATC.DSRVEC')
  SubSystem1899 : Subsystem  TreatAsAtomicUnit=on  mask(***ATC.DSRVEC***){bd_version=1.01.A, r0=DSM11, r1=-1, r2=, r3=, r4=, r5=, r6=, r7=, r8=, r9=, c0=, c1=, c2=, c3=, c4=, c5=, c6=, c7=, c8=, c9=}
      mask prompts: bd_version "Version"
      mask help: これはAutoCからのブロックです．編集禁止．
      settings: OpenFcn=warndlg('変更禁止!');
      mask Help: web(ui_blockhelpmng(gcb));
      mask Display: disp('ATC.DSRVEC')
  Block5202 : Outport
  wires:
  Block5200/1 -> SubSystem1899/1(element), SubSystem1898/1(element), SubSystem1897/1(element)
  SubSystem1899/1(Output) -> Block5201/4
  SubSystem1898/1(Output) -> Block5201/3
  SubSystem1897/1(Output) -> Block5201/2
  Block5201/1 -> Block5202/1
  Block5199/1 -> Block5201/1

== system lib_all/SubSystem1879/SubSystem1887/SubSystem1900/SubSystem1901 ==
  note: ***編集禁止***
  element : Inport
  Inside_DSR : DataStoreRead  DataStoreName=DSM4
  Selector : Selector  IndexOptions=Index vector (port)  Indices=[1 3]  OutputSizes=1
  Output : Outport
  wires:
  Selector/1 -> Output/1
  element/1 -> Selector/2
  Inside_DSR/1 -> Selector/1

== system lib_all/SubSystem1879/SubSystem1887/SubSystem1900/SubSystem1902 ==
  Block5210 : Inport
  Block5211 : Inport  Port=2
  Block5212 : MultiPortSwitch  zeroidx=off  RndMeth=Zero  SaturateOnIntegerOverflow=on  InputSameDT=on
  SubSystem1903 : Subsystem  TreatAsAtomicUnit=on  mask(***ATC.DSRVEC***){bd_version=1.01.A, r0=DSM18, r1=-1, r2=, r3=, r4=, r5=, r6=, r7=, r8=, r9=, c0=, c1=, c2=, c3=, c4=, c5=, c6=, c7=, c8=, c9=}
      mask prompts: bd_version "Version"
      mask help: これはAutoCからのブロックです．編集禁止．
      settings: OpenFcn=warndlg('変更禁止!');
      mask Help: web(ui_blockhelpmng(gcb));
      mask Display: disp('ATC.DSRVEC')
  SubSystem1904 : Subsystem  TreatAsAtomicUnit=on  mask(***ATC.DSRVEC***){bd_version=1.01.A, r0=DSM8, r1=-1, r2=, r3=, r4=, r5=, r6=, r7=, r8=, r9=, c0=, c1=, c2=, c3=, c4=, c5=, c6=, c7=, c8=, c9=}
      mask prompts: bd_version "Version"
      mask help: これはAutoCからのブロックです．編集禁止．
      settings: OpenFcn=warndlg('変更禁止!');
      mask Help: web(ui_blockhelpmng(gcb));
      mask Display: disp('ATC.DSRVEC')
  SubSystem1905 : Subsystem  TreatAsAtomicUnit=on  mask(***ATC.DSRVEC***){bd_version=1.01.A, r0=DSM11, r1=-1, r2=, r3=, r4=, r5=, r6=, r7=, r8=, r9=, c0=, c1=, c2=, c3=, c4=, c5=, c6=, c7=, c8=, c9=}
      mask prompts: bd_version "Version"
      mask help: これはAutoCからのブロックです．編集禁止．
      settings: OpenFcn=warndlg('変更禁止!');
      mask Help: web(ui_blockhelpmng(gcb));
      mask Display: disp('ATC.DSRVEC')
  Block5213 : Outport
  wires:
  Block5211/1 -> SubSystem1905/1(element), SubSystem1904/1(element), SubSystem1903/1(element)
  SubSystem1905/1(Output) -> Block5212/4
  SubSystem1904/1(Output) -> Block5212/3
  SubSystem1903/1(Output) -> Block5212/2
  Block5212/1 -> Block5213/1
  Block5210/1 -> Block5212/1

== system lib_all/SubSystem1879/SubSystem1887/SubSystem1900/SubSystem1906 ==
  Block5214 : Inport
  Block5215 : Inport  Port=2
  Block5216 : MultiPortSwitch  zeroidx=off  RndMeth=Zero  SaturateOnIntegerOverflow=on  InputSameDT=on
  SubSystem1907 : Subsystem  TreatAsAtomicUnit=on  mask(***ATC.DSRVEC***){bd_version=1.01.A, r0=DSM18, r1=-1, r2=, r3=, r4=, r5=, r6=, r7=, r8=, r9=, c0=, c1=, c2=, c3=, c4=, c5=, c6=, c7=, c8=, c9=}
      mask prompts: bd_version "Version"
      mask help: これはAutoCからのブロックです．編集禁止．
      settings: OpenFcn=warndlg('変更禁止!');
      mask Help: web(ui_blockhelpmng(gcb));
      mask Display: disp('ATC.DSRVEC')
  SubSystem1908 : Subsystem  TreatAsAtomicUnit=on  mask(***ATC.DSRVEC***){bd_version=1.01.A, r0=DSM8, r1=-1, r2=, r3=, r4=, r5=, r6=, r7=, r8=, r9=, c0=, c1=, c2=, c3=, c4=, c5=, c6=, c7=, c8=, c9=}
      mask prompts: bd_version "Version"
      mask help: これはAutoCからのブロックです．編集禁止．
      settings: OpenFcn=warndlg('変更禁止!');
      mask Help: web(ui_blockhelpmng(gcb));
      mask Display: disp('ATC.DSRVEC')
  SubSystem1909 : Subsystem  TreatAsAtomicUnit=on  mask(***ATC.DSRVEC***){bd_version=1.01.A, r0=DSM11, r1=-1, r2=, r3=, r4=, r5=, r6=, r7=, r8=, r9=, c0=, c1=, c2=, c3=, c4=, c5=, c6=, c7=, c8=, c9=}
      mask prompts: bd_version "Version"
      mask help: これはAutoCからのブロックです．編集禁止．
      settings: OpenFcn=warndlg('変更禁止!');
      mask Help: web(ui_blockhelpmng(gcb));
      mask Display: disp('ATC.DSRVEC')
  Block5217 : Outport
  wires:
  Block5215/1 -> SubSystem1909/1(element), SubSystem1908/1(element), SubSystem1907/1(element)
  SubSystem1909/1(Output) -> Block5216/4
  SubSystem1908/1(Output) -> Block5216/3
  SubSystem1907/1(Output) -> Block5216/2
  Block5216/1 -> Block5217/1
  Block5214/1 -> Block5216/1

== system lib_all/SubSystem1879/SubSystem1887/SubSystem1910/SubSystem1911 ==
  note: ***編集禁止***
  element : Inport
  Inside_DSR : DataStoreRead  DataStoreName=DSM2
  Selector : Selector  IndexOptions=Index vector (port)  Indices=[1 3]  OutputSizes=1
  Output : Outport
  wires:
  Selector/1 -> Output/1
  element/1 -> Selector/2
  Inside_DSR/1 -> Selector/1

== system lib_all/SubSystem1879/SubSystem1887/SubSystem1910/SubSystem1912 ==
  Block5225 : Inport
  Block5226 : Inport  Port=2
  Block5227 : MultiPortSwitch  zeroidx=off  RndMeth=Zero  SaturateOnIntegerOverflow=on  InputSameDT=on
  SubSystem1913 : Subsystem  TreatAsAtomicUnit=on  mask(***ATC.DSRVEC***){bd_version=1.01.A, r0=DSM18, r1=-1, r2=, r3=, r4=, r5=, r6=, r7=, r8=, r9=, c0=, c1=, c2=, c3=, c4=, c5=, c6=, c7=, c8=, c9=}
      mask prompts: bd_version "Version"
      mask help: これはAutoCからのブロックです．編集禁止．
      settings: OpenFcn=warndlg('変更禁止!');
      mask Help: web(ui_blockhelpmng(gcb));
      mask Display: disp('ATC.DSRVEC')
  SubSystem1914 : Subsystem  TreatAsAtomicUnit=on  mask(***ATC.DSRVEC***){bd_version=1.01.A, r0=DSM8, r1=-1, r2=, r3=, r4=, r5=, r6=, r7=, r8=, r9=, c0=, c1=, c2=, c3=, c4=, c5=, c6=, c7=, c8=, c9=}
      mask prompts: bd_version "Version"
      mask help: これはAutoCからのブロックです．編集禁止．
      settings: OpenFcn=warndlg('変更禁止!');
      mask Help: web(ui_blockhelpmng(gcb));
      mask Display: disp('ATC.DSRVEC')
  SubSystem1915 : Subsystem  TreatAsAtomicUnit=on  mask(***ATC.DSRVEC***){bd_version=1.01.A, r0=DSM11, r1=-1, r2=, r3=, r4=, r5=, r6=, r7=, r8=, r9=, c0=, c1=, c2=, c3=, c4=, c5=, c6=, c7=, c8=, c9=}
      mask prompts: bd_version "Version"
      mask help: これはAutoCからのブロックです．編集禁止．
      settings: OpenFcn=warndlg('変更禁止!');
      mask Help: web(ui_blockhelpmng(gcb));
      mask Display: disp('ATC.DSRVEC')
  Block5228 : Outport
  wires:
  Block5226/1 -> SubSystem1915/1(element), SubSystem1914/1(element), SubSystem1913/1(element)
  SubSystem1915/1(Output) -> Block5227/4
  SubSystem1914/1(Output) -> Block5227/3
  SubSystem1913/1(Output) -> Block5227/2
  Block5227/1 -> Block5228/1
  Block5225/1 -> Block5227/1

== system lib_all/SubSystem1879/SubSystem1887/SubSystem1910/SubSystem1916 ==
  Block5229 : Inport
  Block5230 : Inport  Port=2
  Block5231 : MultiPortSwitch  zeroidx=off  RndMeth=Zero  SaturateOnIntegerOverflow=on  InputSameDT=on
  SubSystem1917 : Subsystem  TreatAsAtomicUnit=on  mask(***ATC.DSRVEC***){bd_version=1.01.A, r0=DSM18, r1=-1, r2=, r3=, r4=, r5=, r6=, r7=, r8=, r9=, c0=, c1=, c2=, c3=, c4=, c5=, c6=, c7=, c8=, c9=}
      mask prompts: bd_version "Version"
      mask help: これはAutoCからのブロックです．編集禁止．
      settings: OpenFcn=warndlg('変更禁止!');
      mask Help: web(ui_blockhelpmng(gcb));
      mask Display: disp('ATC.DSRVEC')
  SubSystem1918 : Subsystem  TreatAsAtomicUnit=on  mask(***ATC.DSRVEC***){bd_version=1.01.A, r0=DSM8, r1=-1, r2=, r3=, r4=, r5=, r6=, r7=, r8=, r9=, c0=, c1=, c2=, c3=, c4=, c5=, c6=, c7=, c8=, c9=}
      mask prompts: bd_version "Version"
      mask help: これはAutoCからのブロックです．編集禁止．
      settings: OpenFcn=warndlg('変更禁止!');
      mask Help: web(ui_blockhelpmng(gcb));
      mask Display: disp('ATC.DSRVEC')
  SubSystem1919 : Subsystem  TreatAsAtomicUnit=on  mask(***ATC.DSRVEC***){bd_version=1.01.A, r0=DSM11, r1=-1, r2=, r3=, r4=, r5=, r6=, r7=, r8=, r9=, c0=, c1=, c2=, c3=, c4=, c5=, c6=, c7=, c8=, c9=}
      mask prompts: bd_version "Version"
      mask help: これはAutoCからのブロックです．編集禁止．
      settings: OpenFcn=warndlg('変更禁止!');
      mask Help: web(ui_blockhelpmng(gcb));
      mask Display: disp('ATC.DSRVEC')
  Block5232 : Outport
  wires:
  Block5230/1 -> SubSystem1919/1(element), SubSystem1918/1(element), SubSystem1917/1(element)
  SubSystem1919/1(Output) -> Block5231/4
  SubSystem1918/1(Output) -> Block5231/3
  SubSystem1917/1(Output) -> Block5231/2
  Block5231/1 -> Block5232/1
  Block5229/1 -> Block5231/1

== system lib_all/SubSystem1920/SubSystem1922/SubSystem1923/Product ==
  note: ***編集禁止***
  mult : Inport
  div : Inport  Port=2
  init : Inport  Port=3
  Constant1 : Constant  OutDataTypeStr=Inherit: Inherit via back propagation
  Constant2 : Constant  Value=0  OutDataTypeStr=Inherit: Inherit via back propagation
  Product : Product  Inputs=*/  SaturateOnIntegerOverflow=on  RndMeth=Zero  InputSameDT=on  OutDataTypeStr=Inherit: Same as first input
  Relational Operator : RelationalOperator  Operator=~=  InputSameDT=on
  Switch : Switch  Threshold=0.5  SaturateOnIntegerOverflow=on  InputSameDT=on
  Switch1 : Switch  Threshold=0.5  SaturateOnIntegerOverflow=on  InputSameDT=on
  Out : Outport
  wires:
  mult/1 -> Product/1
  Product/1 -> Switch/1
  Relational Operator/1 -> Switch/2, Switch1/2
  init/1 -> Switch/3
  Switch/1 -> Out/1
  div/1 -> Switch1/1, Relational Operator/1
  Switch1/1 -> Product/2
  Constant2/1 -> Relational Operator/2
  Constant1/1 -> Switch1/3

== system lib_all/SubSystem1920/SubSystem1922/SubSystem1925/SubSystem1926 ==
  note: ***編集禁止***
  In1 : Inport
  In2 : Inport  Port=2
  RO1 : RelationalOperator  Operator=>  InputSameDT=on
  single1 : DataTypeConversion  OutDataTypeStr=single  SaturateOnIntegerOverflow=on  RndMeth=Zero
  single2 : DataTypeConversion  OutDataTypeStr=single  SaturateOnIntegerOverflow=on  RndMeth=Zero
  Out1 : Outport
  wires:
  single2/1 -> RO1/2
  single1/1 -> RO1/1
  In1/1 -> single1/1
  In2/1 -> single2/1
  RO1/1 -> Out1/1

== system lib_all/SubSystem1920/SubSystem1922/SubSystem1925/SubSystem1927 ==
  note: ***編集禁止***
  In1 : Inport
  In2 : Inport  Port=2
  LO1 : Logic
  RO1 : RelationalOperator  Operator=<  InputSameDT=on
  RO2 : RelationalOperator  Operator=~=  InputSameDT=on
  single1 : DataTypeConversion  OutDataTypeStr=single  SaturateOnIntegerOverflow=on  RndMeth=Zero
  single2 : DataTypeConversion  OutDataTypeStr=single  SaturateOnIntegerOverflow=on  RndMeth=Zero
  Out1 : Outport
  wires:
  LO1/1 -> Out1/1
  RO2/1 -> LO1/2
  RO1/1 -> LO1/1
  single2/1 -> RO2/2, RO1/2
  single1/1 -> RO2/1, RO1/1
  In1/1 -> single1/1
  In2/1 -> single2/1

== system lib_all/SubSystem1920/SubSystem1928/SubSystem1932/SubSystem1933 ==
  Block5295 : Inport
  Block5296 : Inport  Port=2
  Block5297 : MultiPortSwitch  zeroidx=off  RndMeth=Zero  SaturateOnIntegerOverflow=on  InputSameDT=on
  SubSystem1934 : Subsystem  TreatAsAtomicUnit=on  mask(***ATC.DSRVEC***){bd_version=1.01.A, r0=DSM18, r1=-1, r2=, r3=, r4=, r5=, r6=, r7=, r8=, r9=, c0=, c1=, c2=, c3=, c4=, c5=, c6=, c7=, c8=, c9=}
      mask prompts: bd_version "Version"
      mask help: これはAutoCからのブロックです．編集禁止．
      settings: OpenFcn=warndlg('変更禁止!');
      mask Help: web(ui_blockhelpmng(gcb));
      mask Display: disp('ATC.DSRVEC')
  SubSystem1935 : Subsystem  TreatAsAtomicUnit=on  mask(***ATC.DSRVEC***){bd_version=1.01.A, r0=DSM8, r1=-1, r2=, r3=, r4=, r5=, r6=, r7=, r8=, r9=, c0=, c1=, c2=, c3=, c4=, c5=, c6=, c7=, c8=, c9=}
      mask prompts: bd_version "Version"
      mask help: これはAutoCからのブロックです．編集禁止．
      settings: OpenFcn=warndlg('変更禁止!');
      mask Help: web(ui_blockhelpmng(gcb));
      mask Display: disp('ATC.DSRVEC')
  SubSystem1936 : Subsystem  TreatAsAtomicUnit=on  mask(***ATC.DSRVEC***){bd_version=1.01.A, r0=DSM11, r1=-1, r2=, r3=, r4=, r5=, r6=, r7=, r8=, r9=, c0=, c1=, c2=, c3=, c4=, c5=, c6=, c7=, c8=, c9=}
      mask prompts: bd_version "Version"
      mask help: これはAutoCからのブロックです．編集禁止．
      settings: OpenFcn=warndlg('変更禁止!');
      mask Help: web(ui_blockhelpmng(gcb));
      mask Display: disp('ATC.DSRVEC')
  Block5298 : Outport
  wires:
  Block5296/1 -> SubSystem1936/1(element), SubSystem1935/1(element), SubSystem1934/1(element)
  SubSystem1936/1(Output) -> Block5297/4
  SubSystem1935/1(Output) -> Block5297/3
  SubSystem1934/1(Output) -> Block5297/2
  Block5297/1 -> Block5298/1
  Block5295/1 -> Block5297/1

== system lib_all/SubSystem1920/SubSystem1928/SubSystem1932/SubSystem1937 ==
  Block5299 : Inport
  Block5300 : Inport  Port=2
  Block5301 : MultiPortSwitch  zeroidx=off  RndMeth=Zero  SaturateOnIntegerOverflow=on  InputSameDT=on
  SubSystem1938 : Subsystem  TreatAsAtomicUnit=on  mask(***ATC.DSRVEC***){bd_version=1.01.A, r0=DSM18, r1=-1, r2=, r3=, r4=, r5=, r6=, r7=, r8=, r9=, c0=, c1=, c2=, c3=, c4=, c5=, c6=, c7=, c8=, c9=}
      mask prompts: bd_version "Version"
      mask help: これはAutoCからのブロックです．編集禁止．
      settings: OpenFcn=warndlg('変更禁止!');
      mask Help: web(ui_blockhelpmng(gcb));
      mask Display: disp('ATC.DSRVEC')
  SubSystem1939 : Subsystem  TreatAsAtomicUnit=on  mask(***ATC.DSRVEC***){bd_version=1.01.A, r0=DSM8, r1=-1, r2=, r3=, r4=, r5=, r6=, r7=, r8=, r9=, c0=, c1=, c2=, c3=, c4=, c5=, c6=, c7=, c8=, c9=}
      mask prompts: bd_version "Version"
      mask help: これはAutoCからのブロックです．編集禁止．
      settings: OpenFcn=warndlg('変更禁止!');
      mask Help: web(ui_blockhelpmng(gcb));
      mask Display: disp('ATC.DSRVEC')
  SubSystem1940 : Subsystem  TreatAsAtomicUnit=on  mask(***ATC.DSRVEC***){bd_version=1.01.A, r0=DSM11, r1=-1, r2=, r3=, r4=, r5=, r6=, r7=, r8=, r9=, c0=, c1=, c2=, c3=, c4=, c5=, c6=, c7=, c8=, c9=}
      mask prompts: bd_version "Version"
      mask help: これはAutoCからのブロックです．編集禁止．
      settings: OpenFcn=warndlg('変更禁止!');
      mask Help: web(ui_blockhelpmng(gcb));
      mask Display: disp('ATC.DSRVEC')
  Block5302 : Outport
  wires:
  Block5300/1 -> SubSystem1940/1(element), SubSystem1939/1(element), SubSystem1938/1(element)
  SubSystem1940/1(Output) -> Block5301/4
  SubSystem1939/1(Output) -> Block5301/3
  SubSystem1938/1(Output) -> Block5301/2
  Block5301/1 -> Block5302/1
  Block5299/1 -> Block5301/1

== system lib_all/SubSystem1920/SubSystem1928/SubSystem1941/SubSystem1942 ==
  note: ***編集禁止***
  element : Inport
  Inside_DSR : DataStoreRead  DataStoreName=DSM4
  Selector : Selector  IndexOptions=Index vector (port)  Indices=[1 3]  OutputSizes=1
  Output : Outport
  wires:
  Inside_DSR/1 -> Selector/1
  element/1 -> Selector/2
  Selector/1 -> Output/1

== system lib_all/SubSystem1920/SubSystem1928/SubSystem1941/SubSystem1943 ==
  Block5310 : Inport
  Block5311 : Inport  Port=2
  Block5312 : MultiPortSwitch  zeroidx=off  RndMeth=Zero  SaturateOnIntegerOverflow=on  InputSameDT=on
  SubSystem1944 : Subsystem  TreatAsAtomicUnit=on  mask(***ATC.DSRVEC***){bd_version=1.01.A, r0=DSM18, r1=-1, r2=, r3=, r4=, r5=, r6=, r7=, r8=, r9=, c0=, c1=, c2=, c3=, c4=, c5=, c6=, c7=, c8=, c9=}
      mask prompts: bd_version "Version"
      mask help: これはAutoCからのブロックです．編集禁止．
      settings: OpenFcn=warndlg('変更禁止!');
      mask Help: web(ui_blockhelpmng(gcb));
      mask Display: disp('ATC.DSRVEC')
  SubSystem1945 : Subsystem  TreatAsAtomicUnit=on  mask(***ATC.DSRVEC***){bd_version=1.01.A, r0=DSM8, r1=-1, r2=, r3=, r4=, r5=, r6=, r7=, r8=, r9=, c0=, c1=, c2=, c3=, c4=, c5=, c6=, c7=, c8=, c9=}
      mask prompts: bd_version "Version"
      mask help: これはAutoCからのブロックです．編集禁止．
      settings: OpenFcn=warndlg('変更禁止!');
      mask Help: web(ui_blockhelpmng(gcb));
      mask Display: disp('ATC.DSRVEC')
  SubSystem1946 : Subsystem  TreatAsAtomicUnit=on  mask(***ATC.DSRVEC***){bd_version=1.01.A, r0=DSM11, r1=-1, r2=, r3=, r4=, r5=, r6=, r7=, r8=, r9=, c0=, c1=, c2=, c3=, c4=, c5=, c6=, c7=, c8=, c9=}
      mask prompts: bd_version "Version"
      mask help: これはAutoCからのブロックです．編集禁止．
      settings: OpenFcn=warndlg('変更禁止!');
      mask Help: web(ui_blockhelpmng(gcb));
      mask Display: disp('ATC.DSRVEC')
  Block5313 : Outport
  wires:
  Block5311/1 -> SubSystem1946/1(element), SubSystem1945/1(element), SubSystem1944/1(element)
  SubSystem1946/1(Output) -> Block5312/4
  SubSystem1945/1(Output) -> Block5312/3
  SubSystem1944/1(Output) -> Block5312/2
  Block5312/1 -> Block5313/1
  Block5310/1 -> Block5312/1
